simulink model "TosAeb"  (R2021a)
settings: Solver=FixedStepDiscrete  StartTime=0.0  StopTime=inf  FixedStep=0.01  RelTol=1e-3
undefined symbols (not defined in the file or in its folder): ACCModSts, AccSts1, AEB_ObjectMovingOrientation, AEB_ObjectMovingState, CN_BTCActive, CN_CurrentGearR, CN_CurrentGearR_DHT, CN_PTCActive, CN_VDCActive, CN_VSC_EspOff, CN_VSC_FCW_AEB_Error, CN_VSC_FCW_AEB_NoDispay, CN_VSC_FCW_AEB_PerfmDgdtn, CN_VSC_FCW_AEB_TempUnAvlbl, CN_VSC_VehStandstill, CNaccelAvoidMax, CNaccelAvoidMin, CNbrakePedalPress, CNcomfortStrLeftAccelMax, CNcomfortStrLeftAccelMin, CNcomfortStrRightAccelMax, CNcomfortStrRightAccelMin, CNDrvDoorOpen, CNDrvModeEco, CNDrvModeNorm, CNDrvModeOther, CNDrvModeSport, CNDrvSBRreminder, CNNull, CNobjInvalidID, CNPassDoorOpen, CNPassSBRreminder, CNPEBSAccelNegInf, CNPEBSAccelNull, CNPEBSTimeInf, CNPEBSTimeNull, CNSTOPMAX, CNSTOPNULL, CNsubjWidth, CNSwtOFF, CNSwtON, CNTTCMAX, CNTTCNULL, CNVehConfigAEBCycNone, CNVehConfigAEBNone, CNVehConfigAEBPedNone, CNVehConfigFCWNone, CNVehConfigJANone, CollisionSeverity, FALSE, FCWSnvty, JACycObjCalcInfoDefaultParams, JAPedObjCalcInfoDefaultParams, JAVehObjCalcInfoDefaultParams, latCycObjCalcInfoDefaultParams, latPedObjCalcInfoDefaultParams, longObjCalcInfoDefaultParams, ModSts, movingStatus, ObjectType ... (+1112)
libraries: simulink x3031, AEBSFunctionLib x50, Ta_Library x33, AEBSStateMachineLib x23, Mylibrary x12, simulink_extras x7, ObjSelect_lib x3, dspstat3 x2
(part 45 of 102)

== system TosAeb/FcwAebJa_Step_sys/ALG_AEBSystem/ALG_AEBController/AEB_FuncImpManagementApp/FuncImpArbCalc/BrkFuncImpArbCalc/BrkFuncImpSuppCondCalc/AEBpartFuncImpSuppCondCalc ==
  AEBfullModStsArb : Inport
  pedAEBfullModStsArb : Inport  Port=2
  cycAEBpartModStsArb : Inport  Port=3
  cycAEBfullModStsArb : Inport  Port=4
  JA_vehAEBfullModStsArb : Inport  Port=5
  EBAModStsArb : Inport  Port=6
  JA_PTW_AEBfullModStsArb : Inport  Port=7
  JA_cycAEBfullModStsArb : Inport  Port=8
  JA_pedAEBfullModStsArb : Inport  Port=9
  Compare To Constant{1..3,7..12} (9 alike) : simulink/Logic and Bit Operations/Compare To Constant  relop===  const=ModSts.ActiveState  OutDataTypeStr=boolean
  Logical Operator1 : Logic  Operator=OR  Inputs=9  AllPortsSameDT=off  OutDataTypeStr=boolean
  AEBpartFuncImpSuppCond : Outport
  wires:
  Compare To Constant3/1 -> Logical Operator1/9
  Compare To Constant2/1 -> Logical Operator1/8
  Compare To Constant1/1 -> Logical Operator1/7
  JA_pedAEBfullModStsArb/1 -> Compare To Constant3/1
  JA_cycAEBfullModStsArb/1 -> Compare To Constant2/1
  JA_PTW_AEBfullModStsArb/1 -> Compare To Constant1/1
  Logical Operator1/1 -> AEBpartFuncImpSuppCond/1
  Compare To Constant9/1 -> Logical Operator1/3
  Compare To Constant11/1 -> Logical Operator1/5
  pedAEBfullModStsArb/1 -> Compare To Constant8/1
  Compare To Constant10/1 -> Logical Operator1/4
  Compare To Constant7/1 -> Logical Operator1/1
  Compare To Constant8/1 -> Logical Operator1/2
  EBAModStsArb/1 -> Compare To Constant12/1
  AEBfullModStsArb/1 -> Compare To Constant7/1
  cycAEBpartModStsArb/1 -> Compare To Constant9/1
  cycAEBfullModStsArb/1 -> Compare To Constant10/1
  JA_vehAEBfullModStsArb/1 -> Compare To Constant11/1
  Compare To Constant12/1 -> Logical Operator1/6

== system TosAeb/FcwAebJa_Step_sys/ALG_AEBSystem/ALG_AEBController/AEB_FuncImpManagementApp/FuncImpArbCalc/BrkFuncImpArbCalc/BrkFuncImpSuppCondCalc/EBAFuncImpSuppCondCalc ==
  Constant : Constant  Value=FALSE
  EBAFuncImpSuppCond : Outport
  wires:
  Constant/1 -> EBAFuncImpSuppCond/1

== system TosAeb/FcwAebJa_Step_sys/ALG_AEBSystem/ALG_AEBController/AEB_FuncImpManagementApp/FuncImpArbCalc/BrkFuncImpArbCalc/BrkFuncImpSuppCondCalc/FCTBFuncImpSuppCondCalc ==
  AEBpartModStsArb : Inport
  AEBfullModStsArb : Inport  Port=2
  EBAModStsArb : Inport  Port=3
  pedAEBfullModStsArb : Inport  Port=4
  cycAEBfullModStsArb : Inport  Port=5
  cycAEBpartModStsArb : Inport  Port=6
  JA_vehAEBfullModStsArb : Inport  Port=7
  JA_PTW_AEBModStsArb : Inport  Port=8
  JA_cycAEBModStsArb : Inport  Port=9
  JA_pedAEBModStsArb : Inport  Port=10
  Compare To Constant{,1..9} (10 alike) : simulink/Logic and Bit Operations/Compare To Constant  relop===  const=ModSts.ActiveState  OutDataTypeStr=boolean
  Logical Operator : Logic  Operator=OR  Inputs=10  AllPortsSameDT=off  OutDataTypeStr=boolean
  FCTBFuncImpSuppCond : Outport
  wires:
  JA_pedAEBModStsArb/1 -> Compare To Constant9/1
  Compare To Constant9/1 -> Logical Operator/10
  JA_cycAEBModStsArb/1 -> Compare To Constant8/1
  Compare To Constant8/1 -> Logical Operator/9
  Compare To Constant7/1 -> Logical Operator/8
  JA_PTW_AEBModStsArb/1 -> Compare To Constant7/1
  Compare To Constant2/1 -> Logical Operator/3
  Compare To Constant5/1 -> Logical Operator/6
  Compare To Constant/1 -> Logical Operator/1
  Compare To Constant4/1 -> Logical Operator/5
  EBAModStsArb/1 -> Compare To Constant2/1
  Compare To Constant6/1 -> Logical Operator/7
  Compare To Constant3/1 -> Logical Operator/4
  Logical Operator/1 -> FCTBFuncImpSuppCond/1
  Compare To Constant1/1 -> Logical Operator/2
  pedAEBfullModStsArb/1 -> Compare To Constant3/1
  cycAEBfullModStsArb/1 -> Compare To Constant4/1
  AEBfullModStsArb/1 -> Compare To Constant1/1
  AEBpartModStsArb/1 -> Compare To Constant/1
  JA_vehAEBfullModStsArb/1 -> Compare To Constant6/1
  cycAEBpartModStsArb/1 -> Compare To Constant5/1

== system TosAeb/FcwAebJa_Step_sys/ALG_AEBSystem/ALG_AEBController/AEB_FuncImpManagementApp/FuncImpArbCalc/BrkFuncImpArbCalc/BrkFuncImpSuppCondCalc/JA_PTW_AEBfullFuncImpSuppCondCalc ==
  AEBfullModStsArb : Inport
  pedAEBfullModStsArb : Inport  Port=2
  JA_pedAEBfullModStsArb : Inport  Port=3
  cycAEBfullModStsArb : Inport  Port=4
  EBAModStsArb : Inport  Port=5
  Compare To Constant{7..10,12} (5 alike) : simulink/Logic and Bit Operations/Compare To Constant  relop===  const=ModSts.ActiveState  OutDataTypeStr=boolean
  Logical Operator1 : Logic  Operator=OR  Inputs=5  AllPortsSameDT=off  OutDataTypeStr=boolean
  JA_PTW_AEBfullFuncImpSuppCond : Outport
  wires:
  Compare To Constant12/1 -> Logical Operator1/5
  cycAEBfullModStsArb/1 -> Compare To Constant10/1
  JA_pedAEBfullModStsArb/1 -> Compare To Constant9/1
  AEBfullModStsArb/1 -> Compare To Constant7/1
  EBAModStsArb/1 -> Compare To Constant12/1
  Compare To Constant8/1 -> Logical Operator1/2
  Compare To Constant7/1 -> Logical Operator1/1
  Compare To Constant10/1 -> Logical Operator1/4
  pedAEBfullModStsArb/1 -> Compare To Constant8/1
  Compare To Constant9/1 -> Logical Operator1/3
  Logical Operator1/1 -> JA_PTW_AEBfullFuncImpSuppCond/1

== system TosAeb/FcwAebJa_Step_sys/ALG_AEBSystem/ALG_AEBController/AEB_FuncImpManagementApp/FuncImpArbCalc/BrkFuncImpArbCalc/BrkFuncImpSuppCondCalc/JA_cycAEBfullFuncImpSuppCondCalc ==
  cycAEBfullModStsArb : Inport
  pedAEBfullModStsArb : Inport  Port=2
  EBAModStsArb : Inport  Port=3
  JA_pedAEBModStsArb : Inport  Port=4
  Compare To Constant{1,19..21} (4 alike) : simulink/Logic and Bit Operations/Compare To Constant  relop===  const=ModSts.ActiveState  OutDataTypeStr=boolean
  Logical Operator3 : Logic  Operator=OR  Inputs=4  AllPortsSameDT=off  OutDataTypeStr=boolean
  JA_cycAEBfullFuncImpSuppCond : Outport
  wires:
  Compare To Constant1/1 -> Logical Operator3/4
  JA_pedAEBModStsArb/1 -> Compare To Constant1/1
  Compare To Constant19/1 -> Logical Operator3/1
  Compare To Constant21/1 -> Logical Operator3/3
  Compare To Constant20/1 -> Logical Operator3/2
  pedAEBfullModStsArb/1 -> Compare To Constant20/1
  EBAModStsArb/1 -> Compare To Constant21/1
  Logical Operator3/1 -> JA_cycAEBfullFuncImpSuppCond/1
  cycAEBfullModStsArb/1 -> Compare To Constant19/1

== system TosAeb/FcwAebJa_Step_sys/ALG_AEBSystem/ALG_AEBController/AEB_FuncImpManagementApp/FuncImpArbCalc/BrkFuncImpArbCalc/BrkFuncImpSuppCondCalc/JA_pedAEBfullFuncImpSuppCondCalc ==
  cycAEBfullModStsArb : Inport
  pedAEBfullModStsArb : Inport  Port=2
  EBAModStsArb : Inport  Port=3
  Compare To Constant19 : simulink/Logic and Bit Operations/Compare To Constant  relop===  const=ModSts.ActiveState  OutDataTypeStr=boolean
  Compare To Constant20 : simulink/Logic and Bit Operations/Compare To Constant  relop===  const=ModSts.ActiveState  OutDataTypeStr=boolean
  Compare To Constant21 : simulink/Logic and Bit Operations/Compare To Constant  relop===  const=ModSts.ActiveState  OutDataTypeStr=boolean
  Logical Operator3 : Logic  Operator=OR  Inputs=3  AllPortsSameDT=off  OutDataTypeStr=boolean
  JA_pedAEBfullFuncImpSuppCond : Outport
  wires:
  cycAEBfullModStsArb/1 -> Compare To Constant19/1
  Logical Operator3/1 -> JA_pedAEBfullFuncImpSuppCond/1
  EBAModStsArb/1 -> Compare To Constant21/1
  pedAEBfullModStsArb/1 -> Compare To Constant20/1
  Compare To Constant20/1 -> Logical Operator3/2
  Compare To Constant21/1 -> Logical Operator3/3
  Compare To Constant19/1 -> Logical Operator3/1

== system TosAeb/FcwAebJa_Step_sys/ALG_AEBSystem/ALG_AEBController/AEB_FuncImpManagementApp/FuncImpArbCalc/BrkFuncImpArbCalc/BrkFuncImpSuppCondCalc/JA_vehAEBfullFuncImpSuppCondCalc ==
  AEBfullModStsArb : Inport
  cycAEBfullModStsArb : Inport  Port=2
  pedAEBfullModStsArb : Inport  Port=3
  EBAModStsArb : Inport  Port=4
  JA_PTW_AEBModStsArb : Inport  Port=5
  JA_cycAEBModStsArb : Inport  Port=6
  JA_pedAEBModStsArb : Inport  Port=7
  Compare To Constant{1..3,18..21} (7 alike) : simulink/Logic and Bit Operations/Compare To Constant  relop===  const=ModSts.ActiveState  OutDataTypeStr=boolean
  Logical Operator3 : Logic  Operator=OR  Inputs=7  AllPortsSameDT=off  OutDataTypeStr=boolean
  JA_vehAEBfullFuncImpSuppCond : Outport
  wires:
  Compare To Constant3/1 -> Logical Operator3/7
  Compare To Constant2/1 -> Logical Operator3/6
  Compare To Constant1/1 -> Logical Operator3/5
  JA_pedAEBModStsArb/1 -> Compare To Constant3/1
  JA_cycAEBModStsArb/1 -> Compare To Constant2/1
  JA_PTW_AEBModStsArb/1 -> Compare To Constant1/1
  Compare To Constant19/1 -> Logical Operator3/2
  Compare To Constant21/1 -> Logical Operator3/4
  Compare To Constant20/1 -> Logical Operator3/3
  AEBfullModStsArb/1 -> Compare To Constant18/1
  pedAEBfullModStsArb/1 -> Compare To Constant20/1
  EBAModStsArb/1 -> Compare To Constant21/1
  Logical Operator3/1 -> JA_vehAEBfullFuncImpSuppCond/1
  Compare To Constant18/1 -> Logical Operator3/1
  cycAEBfullModStsArb/1 -> Compare To Constant19/1

== system TosAeb/FcwAebJa_Step_sys/ALG_AEBSystem/ALG_AEBController/AEB_FuncImpManagementApp/FuncImpArbCalc/BrkFuncImpArbCalc/BrkFuncImpSuppCondCalc/cycAEBfullFuncImpSuppCondCalc ==
  pedAEBfullModStsArb : Inport
  EBAModStsArb : Inport  Port=2
  Compare To Constant22 : simulink/Logic and Bit Operations/Compare To Constant  relop===  const=ModSts.ActiveState  OutDataTypeStr=boolean
  Compare To Constant26 : simulink/Logic and Bit Operations/Compare To Constant  relop===  const=ModSts.ActiveState  OutDataTypeStr=boolean
  Logical Operator5 : Logic  Operator=OR  AllPortsSameDT=off  OutDataTypeStr=boolean
  cycAEBfullFuncImpSuppCond : Outport
  wires:
  EBAModStsArb/1 -> Compare To Constant26/1
  Compare To Constant26/1 -> Logical Operator5/2
  Compare To Constant22/1 -> Logical Operator5/1
  pedAEBfullModStsArb/1 -> Compare To Constant22/1
  Logical Operator5/1 -> cycAEBfullFuncImpSuppCond/1

== system TosAeb/FcwAebJa_Step_sys/ALG_AEBSystem/ALG_AEBController/AEB_FuncImpManagementApp/FuncImpArbCalc/BrkFuncImpArbCalc/BrkFuncImpSuppCondCalc/cycAEBpartFuncImpSuppCondCalc ==
  AEBfullModStsArb : Inport
  pedAEBfullModStsArb : Inport  Port=2
  cycAEBfullModStsArb : Inport  Port=3
  JA_vehAEBfullModStsArb : Inport  Port=4
  EBAModStsArb : Inport  Port=5
  JA_PTW_AEBfullModStsArb : Inport  Port=6
  JA_cycAEBfullModStsArb : Inport  Port=7
  JA_pedAEBfullModStsArb : Inport  Port=8
  Compare To Constant{1..3,13..17} (8 alike) : simulink/Logic and Bit Operations/Compare To Constant  relop===  const=ModSts.ActiveState  OutDataTypeStr=boolean
  Logical Operator2 : Logic  Operator=OR  Inputs=8  AllPortsSameDT=off  OutDataTypeStr=boolean
  cycAEBpartFuncImpSuppCond : Outport
  wires:
  Compare To Constant3/1 -> Logical Operator2/8
  Compare To Constant2/1 -> Logical Operator2/7
  Compare To Constant1/1 -> Logical Operator2/6
  JA_pedAEBfullModStsArb/1 -> Compare To Constant3/1
  JA_cycAEBfullModStsArb/1 -> Compare To Constant2/1
  JA_PTW_AEBfullModStsArb/1 -> Compare To Constant1/1
  pedAEBfullModStsArb/1 -> Compare To Constant14/1
  Compare To Constant17/1 -> Logical Operator2/5
  Compare To Constant13/1 -> Logical Operator2/1
  Compare To Constant15/1 -> Logical Operator2/3
  Compare To Constant16/1 -> Logical Operator2/4
  JA_vehAEBfullModStsArb/1 -> Compare To Constant16/1
  Compare To Constant14/1 -> Logical Operator2/2
  Logical Operator2/1 -> cycAEBpartFuncImpSuppCond/1
  EBAModStsArb/1 -> Compare To Constant17/1
  AEBfullModStsArb/1 -> Compare To Constant13/1
  cycAEBfullModStsArb/1 -> Compare To Constant15/1

== system TosAeb/FcwAebJa_Step_sys/ALG_AEBSystem/ALG_AEBController/AEB_FuncImpManagementApp/FuncImpArbCalc/BrkFuncImpArbCalc/BrkFuncImpSuppCondCalc/pedAEBfullFuncImpSuppCondCalc ==
  EBAModStsArb : Inport
  Compare To Constant27 : simulink/Logic and Bit Operations/Compare To Constant  relop===  const=ModSts.ActiveState  OutDataTypeStr=boolean
  pedAEBfullFuncImpSuppCond : Outport
  wires:
  EBAModStsArb/1 -> Compare To Constant27/1
  Compare To Constant27/1 -> pedAEBfullFuncImpSuppCond/1

== system TosAeb/FcwAebJa_Step_sys/ALG_AEBSystem/ALG_AEBController/AEB_FuncImpManagementApp/FuncImpArbCalc/BrkFuncImpArbCalc/BrkFuncImpSuppCondCalc1/AEBfullFuncImpSuppCondCalc ==
  pedAEBfullModStsArb : Inport
  cycAEBfullModStsArb : Inport  Port=2
  EBAModStsArb : Inport  Port=3
  Compare To Constant23 : simulink/Logic and Bit Operations/Compare To Constant  relop===  const=ModSts.ActiveState  OutDataTypeStr=boolean
  Compare To Constant24 : simulink/Logic and Bit Operations/Compare To Constant  relop===  const=ModSts.ActiveState  OutDataTypeStr=boolean
  Compare To Constant25 : simulink/Logic and Bit Operations/Compare To Constant  relop===  const=ModSts.ActiveState  OutDataTypeStr=boolean
  Logical Operator4 : Logic  Operator=OR  Inputs=3  AllPortsSameDT=off  OutDataTypeStr=boolean
  AEBfullFuncImpSuppCond : Outport
  wires:
  Compare To Constant23/1 -> Logical Operator4/1
  Compare To Constant24/1 -> Logical Operator4/2
  pedAEBfullModStsArb/1 -> Compare To Constant23/1
  EBAModStsArb/1 -> Compare To Constant25/1
  cycAEBfullModStsArb/1 -> Compare To Constant24/1
  Logical Operator4/1 -> AEBfullFuncImpSuppCond/1
  Compare To Constant25/1 -> Logical Operator4/3

== system TosAeb/FcwAebJa_Step_sys/ALG_AEBSystem/ALG_AEBController/AEB_FuncImpManagementApp/FuncImpArbCalc/BrkFuncImpArbCalc/BrkFuncImpSuppCondCalc1/AEBpartFuncImpSuppCondCalc ==
  AEBfullModStsArb : Inport
  pedAEBfullModStsArb : Inport  Port=2
  cycAEBpartModStsArb : Inport  Port=3
  cycAEBfullModStsArb : Inport  Port=4
  JA_vehAEBfullModStsArb : Inport  Port=5
  EBAModStsArb : Inport  Port=6
  Compare To Constant{7..12} (6 alike) : simulink/Logic and Bit Operations/Compare To Constant  relop===  const=ModSts.ActiveState  OutDataTypeStr=boolean
  Logical Operator1 : Logic  Operator=OR  Inputs=6  AllPortsSameDT=off  OutDataTypeStr=boolean
  AEBpartFuncImpSuppCond : Outport
  wires:
  Logical Operator1/1 -> AEBpartFuncImpSuppCond/1
  EBAModStsArb/1 -> Compare To Constant12/1
  Compare To Constant12/1 -> Logical Operator1/6
  Compare To Constant10/1 -> Logical Operator1/4
  AEBfullModStsArb/1 -> Compare To Constant7/1
  Compare To Constant7/1 -> Logical Operator1/1
  pedAEBfullModStsArb/1 -> Compare To Constant8/1
  Compare To Constant8/1 -> Logical Operator1/2
  Compare To Constant9/1 -> Logical Operator1/3
  cycAEBfullModStsArb/1 -> Compare To Constant10/1
  JA_vehAEBfullModStsArb/1 -> Compare To Constant11/1
  cycAEBpartModStsArb/1 -> Compare To Constant9/1
  Compare To Constant11/1 -> Logical Operator1/5

== system TosAeb/FcwAebJa_Step_sys/ALG_AEBSystem/ALG_AEBController/AEB_FuncImpManagementApp/FuncImpArbCalc/BrkFuncImpArbCalc/BrkFuncImpSuppCondCalc1/EBAFuncImpSuppCondCalc ==
  Constant : Constant  Value=FALSE
  EBAFuncImpSuppCond : Outport
  wires:
  Constant/1 -> EBAFuncImpSuppCond/1

== system TosAeb/FcwAebJa_Step_sys/ALG_AEBSystem/ALG_AEBController/AEB_FuncImpManagementApp/FuncImpArbCalc/BrkFuncImpArbCalc/BrkFuncImpSuppCondCalc1/FCTBFuncImpSuppCondCalc ==
  AEBpartModStsArb : Inport
  AEBfullModStsArb : Inport  Port=2
  EBAModStsArb : Inport  Port=3
  pedAEBfullModStsArb : Inport  Port=4
  cycAEBfullModStsArb : Inport  Port=5
  cycAEBpartModStsArb : Inport  Port=6
  JA_vehAEBfullModStsArb : Inport  Port=7
  Compare To Constant{,1..6} (7 alike) : simulink/Logic and Bit Operations/Compare To Constant  relop===  const=ModSts.ActiveState  OutDataTypeStr=boolean
  Logical Operator : Logic  Operator=OR  Inputs=7  AllPortsSameDT=off  OutDataTypeStr=boolean
  FCTBFuncImpSuppCond : Outport
  wires:
  Compare To Constant2/1 -> Logical Operator/3
  Compare To Constant6/1 -> Logical Operator/7
  Compare To Constant3/1 -> Logical Operator/4
  Compare To Constant5/1 -> Logical Operator/6
  Compare To Constant/1 -> Logical Operator/1
  AEBfullModStsArb/1 -> Compare To Constant1/1
  AEBpartModStsArb/1 -> Compare To Constant/1
  Logical Operator/1 -> FCTBFuncImpSuppCond/1
  Compare To Constant4/1 -> Logical Operator/5
  Compare To Constant1/1 -> Logical Operator/2
  EBAModStsArb/1 -> Compare To Constant2/1
  cycAEBfullModStsArb/1 -> Compare To Constant4/1
  pedAEBfullModStsArb/1 -> Compare To Constant3/1
  JA_vehAEBfullModStsArb/1 -> Compare To Constant6/1
  cycAEBpartModStsArb/1 -> Compare To Constant5/1

== system TosAeb/FcwAebJa_Step_sys/ALG_AEBSystem/ALG_AEBController/AEB_FuncImpManagementApp/FuncImpArbCalc/BrkFuncImpArbCalc/BrkFuncImpSuppCondCalc1/JA_vehAEBfullFuncImpSuppCondCalc ==
  AEBfullModStsArb : Inport
  cycAEBfullModStsArb : Inport  Port=2
  pedAEBfullModStsArb : Inport  Port=3
  EBAModStsArb : Inport  Port=4
  Compare To Constant{18..21} (4 alike) : simulink/Logic and Bit Operations/Compare To Constant  relop===  const=ModSts.ActiveState  OutDataTypeStr=boolean
  Logical Operator3 : Logic  Operator=OR  Inputs=4  AllPortsSameDT=off  OutDataTypeStr=boolean
  JA_vehAEBfullFuncImpSuppCond : Outport
  wires:
  Logical Operator3/1 -> JA_vehAEBfullFuncImpSuppCond/1
  AEBfullModStsArb/1 -> Compare To Constant18/1
  cycAEBfullModStsArb/1 -> Compare To Constant19/1
  Compare To Constant19/1 -> Logical Operator3/2
  Compare To Constant20/1 -> Logical Operator3/3
  EBAModStsArb/1 -> Compare To Constant21/1
  pedAEBfullModStsArb/1 -> Compare To Constant20/1
  Compare To Constant21/1 -> Logical Operator3/4
  Compare To Constant18/1 -> Logical Operator3/1

== system TosAeb/FcwAebJa_Step_sys/ALG_AEBSystem/ALG_AEBController/AEB_FuncImpManagementApp/FuncImpArbCalc/BrkFuncImpArbCalc/BrkFuncImpSuppCondCalc1/cycAEBfullFuncImpSuppCondCalc ==
  <pedAEBfullModStsArb> : Inport
  <EBAModStsArb> : Inport  Port=2
  Compare To Constant22 : simulink/Logic and Bit Operations/Compare To Constant  relop===  const=ModSts.ActiveState  OutDataTypeStr=boolean
  Compare To Constant26 : simulink/Logic and Bit Operations/Compare To Constant  relop===  const=ModSts.ActiveState  OutDataTypeStr=boolean
  Logical Operator5 : Logic  Operator=OR  AllPortsSameDT=off  OutDataTypeStr=boolean
  cycAEBfullFuncImpSuppCond1 : Outport
  wires:
  <EBAModStsArb>/1 -> Compare To Constant26/1
  Logical Operator5/1 -> cycAEBfullFuncImpSuppCond1/1
  Compare To Constant22/1 -> Logical Operator5/1
  Compare To Constant26/1 -> Logical Operator5/2
  <pedAEBfullModStsArb>/1 -> Compare To Constant22/1

== system TosAeb/FcwAebJa_Step_sys/ALG_AEBSystem/ALG_AEBController/AEB_FuncImpManagementApp/FuncImpArbCalc/BrkFuncImpArbCalc/BrkFuncImpSuppCondCalc1/cycAEBpartFuncImpSuppCondCalc ==
  <AEBfullModStsArb> : Inport
  <pedAEBfullModStsArb> : Inport  Port=2
  <cycAEBfullModStsArb> : Inport  Port=3
  <JA_vehAEBfullModStsArb> : Inport  Port=4
  <EBAModStsArb> : Inport  Port=5
  Compare To Constant{13..17} (5 alike) : simulink/Logic and Bit Operations/Compare To Constant  relop===  const=ModSts.ActiveState  OutDataTypeStr=boolean
  Logical Operator2 : Logic  Operator=OR  Inputs=5  AllPortsSameDT=off  OutDataTypeStr=boolean
  cycAEBpartFuncImpSuppCond : Outport
  wires:
  Compare To Constant14/1 -> Logical Operator2/2
  Compare To Constant13/1 -> Logical Operator2/1
  Compare To Constant15/1 -> Logical Operator2/3
  Compare To Constant16/1 -> Logical Operator2/4
  Logical Operator2/1 -> cycAEBpartFuncImpSuppCond/1
  <JA_vehAEBfullModStsArb>/1 -> Compare To Constant16/1
  <EBAModStsArb>/1 -> Compare To Constant17/1
  <pedAEBfullModStsArb>/1 -> Compare To Constant14/1
  <AEBfullModStsArb>/1 -> Compare To Constant13/1
  <cycAEBfullModStsArb>/1 -> Compare To Constant15/1
  Compare To Constant17/1 -> Logical Operator2/5

== system TosAeb/FcwAebJa_Step_sys/ALG_AEBSystem/ALG_AEBController/AEB_FuncImpManagementApp/FuncImpArbCalc/BrkFuncImpArbCalc/BrkFuncImpSuppCondCalc1/pedAEBfullFuncImpSuppCondCalc ==
  EBAModStsArb : Inport
  Compare To Constant27 : simulink/Logic and Bit Operations/Compare To Constant  relop===  const=ModSts.ActiveState  OutDataTypeStr=boolean
  pedAEBfullFuncImpSuppCond : Outport
  wires:
  EBAModStsArb/1 -> Compare To Constant27/1
  Compare To Constant27/1 -> pedAEBfullFuncImpSuppCond/1

== system TosAeb/FcwAebJa_Step_sys/ALG_AEBSystem/ALG_AEBController/AEB_FuncImpManagementApp/FuncImpArbCalc/BrkFuncImpArbCalc1/BrkFuncImpAborCondCalc/AEBfullFuncImpAborCondCalc ==
  AEBModStsArb : Inport
  Bus Selector4 : BusSelector  OutputSignals=latAEBModStsArb.pedAEBfullModStsArb,latAEBModStsArb.cycAEBfullModStsArb,vehAEBModStsArb.EBAModStsArb
  Compare To Constant23 : simulink/Logic and Bit Operations/Compare To Constant  relop===  const=ModSts.ActiveState  OutDataTypeStr=boolean
  Compare To Constant24 : simulink/Logic and Bit Operations/Compare To Constant  relop===  const=ModSts.ActiveState  OutDataTypeStr=boolean
  Compare To Constant25 : simulink/Logic and Bit Operations/Compare To Constant  relop===  const=ModSts.ActiveState  OutDataTypeStr=boolean
  Logical Operator4 : Logic  Operator=OR  Inputs=3  AllPortsSameDT=off  OutDataTypeStr=boolean
  AEBfullFuncImpAborCond : Outport
  wires:
  AEBModStsArb/1 -> Bus Selector4/1
  Compare To Constant24/1 -> Logical Operator4/2
  Compare To Constant23/1 -> Logical Operator4/1
  Compare To Constant25/1 -> Logical Operator4/3
  Logical Operator4/1 -> AEBfullFuncImpAborCond/1
  Bus Selector4/1 -> Compare To Constant23/1  "<pedAEBfullModStsArb>"
  Bus Selector4/3 -> Compare To Constant25/1  "<EBAModStsArb>"
  Bus Selector4/2 -> Compare To Constant24/1  "<cycAEBfullModStsArb>"

== system TosAeb/FcwAebJa_Step_sys/ALG_AEBSystem/ALG_AEBController/AEB_FuncImpManagementApp/FuncImpArbCalc/BrkFuncImpArbCalc1/BrkFuncImpAborCondCalc/AEBpartFuncImpAborCondCalc ==
  AEBModStsArb : Inport
  Bus Selector1 : BusSelector  OutputSignals=vehAEBModStsArb.AEBfullModStsArb,latAEBModStsArb.pedAEBfullModStsArb,latAEBModStsArb.cycAEBpartModStsArb,latAEBModStsArb.cycAEBfullModStsArb,JA_vehAEBModStsArb.JA_vehAEBfullModStsArb,vehAEBModStsArb.EBAModStsArb
  Compare To Constant{7..12} (6 alike) : simulink/Logic and Bit Operations/Compare To Constant  relop===  const=ModSts.ActiveState  OutDataTypeStr=boolean
  Logical Operator1 : Logic  Operator=OR  Inputs=6  AllPortsSameDT=off  OutDataTypeStr=boolean
  AEBpartFuncImpAborCond : Outport
  wires:
  AEBModStsArb/1 -> Bus Selector1/1
  Bus Selector1/6 -> Compare To Constant12/1  "<EBAModStsArb>"
  Logical Operator1/1 -> AEBpartFuncImpAborCond/1
  Compare To Constant12/1 -> Logical Operator1/6
  Compare To Constant7/1 -> Logical Operator1/1
  Compare To Constant11/1 -> Logical Operator1/5
  Compare To Constant8/1 -> Logical Operator1/2
  Compare To Constant10/1 -> Logical Operator1/4
  Compare To Constant9/1 -> Logical Operator1/3
  Bus Selector1/5 -> Compare To Constant11/1  "<JA_vehAEBfullModStsArb>"
  Bus Selector1/1 -> Compare To Constant7/1  "<AEBfullModStsArb>"
  Bus Selector1/3 -> Compare To Constant9/1  "<cycAEBpartModStsArb>"
  Bus Selector1/4 -> Compare To Constant10/1  "<cycAEBfullModStsArb>"
  Bus Selector1/2 -> Compare To Constant8/1  "<pedAEBfullModStsArb>"

== system TosAeb/FcwAebJa_Step_sys/ALG_AEBSystem/ALG_AEBController/AEB_FuncImpManagementApp/FuncImpArbCalc/BrkFuncImpArbCalc1/BrkFuncImpAborCondCalc/EBAFuncImpAborCondCalc ==
  Constant : Constant  Value=FALSE
  EBAFuncImpAborCond : Outport
  wires:
  Constant/1 -> EBAFuncImpAborCond/1

== system TosAeb/FcwAebJa_Step_sys/ALG_AEBSystem/ALG_AEBController/AEB_FuncImpManagementApp/FuncImpArbCalc/BrkFuncImpArbCalc1/BrkFuncImpAborCondCalc/FCTBFuncImpAborCondCalc ==
  AEBModStsArb : Inport
  Bus Selector : BusSelector  OutputSignals=vehAEBModStsArb.AEBpartModStsArb,vehAEBModStsArb.AEBfullModStsArb,vehAEBModStsArb.EBAModStsArb,latAEBModStsArb.pedAEBfullModStsArb,latAEBModStsArb.cycAEBfullModStsArb,latAEBModStsArb.cycAEBpartModStsArb,JA_vehAEBModStsArb.JA_vehAEBfullModStsArb
  Compare To Constant{,1..6} (7 alike) : simulink/Logic and Bit Operations/Compare To Constant  relop===  const=ModSts.ActiveState  OutDataTypeStr=boolean
  Logical Operator : Logic  Operator=OR  Inputs=7  AllPortsSameDT=off  OutDataTypeStr=boolean
  FCTBFuncImpAborCond : Outport
  wires:
  AEBModStsArb/1 -> Bus Selector/1
  Bus Selector/3 -> Compare To Constant2/1  "<EBAModStsArb>"
  Bus Selector/4 -> Compare To Constant3/1  "<pedAEBfullModStsArb>"
  Bus Selector/7 -> Compare To Constant6/1  "<JA_vehAEBfullModStsArb>"
  Bus Selector/5 -> Compare To Constant4/1  "<cycAEBfullModStsArb>"
  Bus Selector/6 -> Compare To Constant5/1  "<cycAEBpartModStsArb>"
  Compare To Constant3/1 -> Logical Operator/4
  Compare To Constant/1 -> Logical Operator/1
  Compare To Constant1/1 -> Logical Operator/2
  Compare To Constant6/1 -> Logical Operator/7
  Compare To Constant4/1 -> Logical Operator/5
  Logical Operator/1 -> FCTBFuncImpAborCond/1
  Compare To Constant5/1 -> Logical Operator/6
  Compare To Constant2/1 -> Logical Operator/3
  Bus Selector/2 -> Compare To Constant1/1  "<AEBfullModStsArb>"
  Bus Selector/1 -> Compare To Constant/1  "<AEBpartModStsArb>"

== system TosAeb/FcwAebJa_Step_sys/ALG_AEBSystem/ALG_AEBController/AEB_FuncImpManagementApp/FuncImpArbCalc/BrkFuncImpArbCalc1/BrkFuncImpAborCondCalc/JA_vehAEBfullFuncImpAborCondCalc ==
  AEBModStsArb : Inport
  Bus Selector3 : BusSelector  OutputSignals=vehAEBModStsArb.AEBfullModStsArb,latAEBModStsArb.cycAEBfullModStsArb,latAEBModStsArb.pedAEBfullModStsArb,vehAEBModStsArb.EBAModStsArb
  Compare To Constant{18..21} (4 alike) : simulink/Logic and Bit Operations/Compare To Constant  relop===  const=ModSts.ActiveState  OutDataTypeStr=boolean
  Logical Operator3 : Logic  Operator=OR  Inputs=4  AllPortsSameDT=off  OutDataTypeStr=boolean
  JA_vehAEBfullFuncImpAborCond : Outport
  wires:
  Compare To Constant21/1 -> Logical Operator3/4
  Compare To Constant18/1 -> Logical Operator3/1
  Compare To Constant19/1 -> Logical Operator3/2
  Logical Operator3/1 -> JA_vehAEBfullFuncImpAborCond/1
  Compare To Constant20/1 -> Logical Operator3/3
  Bus Selector3/2 -> Compare To Constant19/1  "<cycAEBfullModStsArb>"
  Bus Selector3/4 -> Compare To Constant21/1  "<EBAModStsArb>"
  Bus Selector3/3 -> Compare To Constant20/1  "<pedAEBfullModStsArb>"
  Bus Selector3/1 -> Compare To Constant18/1  "<AEBfullModStsArb>"
  AEBModStsArb/1 -> Bus Selector3/1

== system TosAeb/FcwAebJa_Step_sys/ALG_AEBSystem/ALG_AEBController/AEB_FuncImpManagementApp/FuncImpArbCalc/BrkFuncImpArbCalc1/BrkFuncImpAborCondCalc/cycAEBfullFuncImpAborCondCalc ==
  AEBModStsArb : Inport
  Bus Selector5 : BusSelector  OutputSignals=latAEBModStsArb.pedAEBfullModStsArb,vehAEBModStsArb.EBAModStsArb
  Compare To Constant22 : simulink/Logic and Bit Operations/Compare To Constant  relop===  const=ModSts.ActiveState  OutDataTypeStr=boolean
  Compare To Constant26 : simulink/Logic and Bit Operations/Compare To Constant  relop===  const=ModSts.ActiveState  OutDataTypeStr=boolean
  Logical Operator5 : Logic  Operator=OR  AllPortsSameDT=off  OutDataTypeStr=boolean
  cycAEBfullFuncImpAborCond : Outport
  wires:
  AEBModStsArb/1 -> Bus Selector5/1
  Bus Selector5/2 -> Compare To Constant26/1  "<EBAModStsArb>"
  Compare To Constant22/1 -> Logical Operator5/1
  Logical Operator5/1 -> cycAEBfullFuncImpAborCond/1
  Bus Selector5/1 -> Compare To Constant22/1  "<pedAEBfullModStsArb>"
  Compare To Constant26/1 -> Logical Operator5/2

== system TosAeb/FcwAebJa_Step_sys/ALG_AEBSystem/ALG_AEBController/AEB_FuncImpManagementApp/FuncImpArbCalc/BrkFuncImpArbCalc1/BrkFuncImpAborCondCalc/cycAEBpartFuncImpAborCondCalc ==
  AEBModStsArb : Inport
  Bus Selector2 : BusSelector  OutputSignals=vehAEBModStsArb.AEBfullModStsArb,latAEBModStsArb.pedAEBfullModStsArb,latAEBModStsArb.cycAEBfullModStsArb,JA_vehAEBModStsArb.JA_vehAEBfullModStsArb,vehAEBModStsArb.EBAModStsArb
  Compare To Constant{13..17} (5 alike) : simulink/Logic and Bit Operations/Compare To Constant  relop===  const=ModSts.ActiveState  OutDataTypeStr=boolean
  Logical Operator2 : Logic  Operator=OR  Inputs=5  AllPortsSameDT=off  OutDataTypeStr=boolean
  cycAEBpartFuncImpAborCond : Outport
  wires:
  Compare To Constant14/1 -> Logical Operator2/2
  Logical Operator2/1 -> cycAEBpartFuncImpAborCond/1
  AEBModStsArb/1 -> Bus Selector2/1
  Bus Selector2/5 -> Compare To Constant17/1  "<EBAModStsArb>"
  Bus Selector2/4 -> Compare To Constant16/1  "<JA_vehAEBfullModStsArb>"
  Compare To Constant13/1 -> Logical Operator2/1
  Compare To Constant15/1 -> Logical Operator2/3
  Compare To Constant16/1 -> Logical Operator2/4
  Compare To Constant17/1 -> Logical Operator2/5
  Bus Selector2/2 -> Compare To Constant14/1  "<pedAEBfullModStsArb>"
  Bus Selector2/3 -> Compare To Constant15/1  "<cycAEBfullModStsArb>"
  Bus Selector2/1 -> Compare To Constant13/1  "<AEBfullModStsArb>"

== system TosAeb/FcwAebJa_Step_sys/ALG_AEBSystem/ALG_AEBController/AEB_FuncImpManagementApp/FuncImpArbCalc/BrkFuncImpArbCalc1/BrkFuncImpAborCondCalc/pedAEBfullFuncImpAborCondCalc ==
  AEBModStsArb : Inport
  Bus Selector6 : BusSelector  OutputSignals=vehAEBModStsArb.EBAModStsArb
  Compare To Constant27 : simulink/Logic and Bit Operations/Compare To Constant  relop===  const=ModSts.ActiveState  OutDataTypeStr=boolean
  pedAEBfullFuncImpAborCond : Outport
  wires:
  AEBModStsArb/1 -> Bus Selector6/1
  Compare To Constant27/1 -> pedAEBfullFuncImpAborCond/1
  Bus Selector6/1 -> Compare To Constant27/1  "<EBAModStsArb>"

== system TosAeb/FcwAebJa_Step_sys/ALG_AEBSystem/ALG_AEBController/AEB_FuncImpManagementApp/FuncImpArbCalc/BrkFuncImpArbCalc1/BrkFuncImpArbCalc/AEBfullFuncImpArbCalc ==
  AEBfullModStsArb : Inport
  AEBfullFuncImpAborCond : Inport  Port=2
  Compare To Constant : simulink/Logic and Bit Operations/Compare To Constant  relop=~=  const=ModSts.ActiveState  OutDataTypeStr=boolean
  Compare To Constant1 : simulink/Logic and Bit Operations/Compare To Constant  relop===  const=FALSE  OutDataTypeStr=boolean
  Constant : Constant  Value=ModSts.StandbyState
  Switch : Switch  Criteria=u2 ~= 0
  Switch1 : Switch  Criteria=u2 ~= 0
  AEBfullFuncImpArb : Outport
  wires:
  AEBfullModStsArb/1 -> Switch1/1, Switch/1, Compare To Constant/1
  Compare To Constant/1 -> Switch/2
  AEBfullFuncImpAborCond/1 -> Compare To Constant1/1
  Compare To Constant1/1 -> Switch1/2
  Constant/1 -> Switch1/3
  Switch1/1 -> Switch/3
  Switch/1 -> AEBfullFuncImpArb/1

== system TosAeb/FcwAebJa_Step_sys/ALG_AEBSystem/ALG_AEBController/AEB_FuncImpManagementApp/FuncImpArbCalc/BrkFuncImpArbCalc1/BrkFuncImpArbCalc/AEBpartFuncImpArbCalc ==
  AEBpartModStsArb : Inport
  AEBpartFuncImpAborCond : Inport  Port=2
  Compare To Constant : simulink/Logic and Bit Operations/Compare To Constant  relop=~=  const=ModSts.ActiveState  OutDataTypeStr=boolean
  Compare To Constant1 : simulink/Logic and Bit Operations/Compare To Constant  relop===  const=FALSE  OutDataTypeStr=boolean
  Constant : Constant  Value=ModSts.StandbyState
  Switch : Switch  Criteria=u2 ~= 0
  Switch1 : Switch  Criteria=u2 ~= 0
  AEBpartFuncImpArb : Outport
  wires:
  AEBpartModStsArb/1 -> Switch1/1, Switch/1, Compare To Constant/1
  Compare To Constant/1 -> Switch/2
  AEBpartFuncImpAborCond/1 -> Compare To Constant1/1
  Compare To Constant1/1 -> Switch1/2
  Constant/1 -> Switch1/3
  Switch1/1 -> Switch/3
  Switch/1 -> AEBpartFuncImpArb/1

== system TosAeb/FcwAebJa_Step_sys/ALG_AEBSystem/ALG_AEBController/AEB_FuncImpManagementApp/FuncImpArbCalc/BrkFuncImpArbCalc1/BrkFuncImpArbCalc/EBAFuncImpArbCalc ==
  EBAModStsArb : Inport
  EBAFuncImpAborCond : Inport  Port=2
  Compare To Constant : simulink/Logic and Bit Operations/Compare To Constant  relop=~=  const=ModSts.ActiveState  OutDataTypeStr=boolean
  Compare To Constant1 : simulink/Logic and Bit Operations/Compare To Constant  relop===  const=FALSE  OutDataTypeStr=boolean
  Constant : Constant  Value=ModSts.StandbyState
  Switch : Switch  Criteria=u2 ~= 0
  Switch1 : Switch  Criteria=u2 ~= 0
  EBAFuncImpArb : Outport
  wires:
  Switch/1 -> EBAFuncImpArb/1
  Switch1/1 -> Switch/3
  Constant/1 -> Switch1/3
  Compare To Constant1/1 -> Switch1/2
  EBAFuncImpAborCond/1 -> Compare To Constant1/1
  Compare To Constant/1 -> Switch/2
  EBAModStsArb/1 -> Compare To Constant/1, Switch/1, Switch1/1

== system TosAeb/FcwAebJa_Step_sys/ALG_AEBSystem/ALG_AEBController/AEB_FuncImpManagementApp/FuncImpArbCalc/BrkFuncImpArbCalc1/BrkFuncImpArbCalc/FCTBFuncImpArbCalc ==
  FCTBModStsArb : Inport
  FCTBFuncImpAborCond : Inport  Port=2
  Compare To Constant : simulink/Logic and Bit Operations/Compare To Constant  relop=~=  const=ModSts.ActiveState  OutDataTypeStr=boolean
  Compare To Constant1 : simulink/Logic and Bit Operations/Compare To Constant  relop===  const=FALSE  OutDataTypeStr=boolean
  Constant : Constant  Value=ModSts.StandbyState
  Switch : Switch  Criteria=u2 ~= 0
  Switch1 : Switch  Criteria=u2 ~= 0
  FCTBFuncImpArb : Outport
  wires:
  FCTBModStsArb/1 -> Switch1/1, Switch/1, Compare To Constant/1
  Compare To Constant/1 -> Switch/2
  FCTBFuncImpAborCond/1 -> Compare To Constant1/1
  Compare To Constant1/1 -> Switch1/2
  Constant/1 -> Switch1/3
  Switch1/1 -> Switch/3
  Switch/1 -> FCTBFuncImpArb/1

== system TosAeb/FcwAebJa_Step_sys/ALG_AEBSystem/ALG_AEBController/AEB_FuncImpManagementApp/FuncImpArbCalc/BrkFuncImpArbCalc1/BrkFuncImpArbCalc/JA_vehAEBfullFuncImpArbCalc ==
  JA_vehAEBfullfullModStsArb : Inport
  JA_vehAEBfullFuncImpAborCond : Inport  Port=2
  Compare To Constant : simulink/Logic and Bit Operations/Compare To Constant  relop=~=  const=ModSts.ActiveState  OutDataTypeStr=boolean
  Compare To Constant1 : simulink/Logic and Bit Operations/Compare To Constant  relop===  const=FALSE  OutDataTypeStr=boolean
  Constant : Constant  Value=ModSts.StandbyState
  Switch : Switch  Criteria=u2 ~= 0
  Switch1 : Switch  Criteria=u2 ~= 0
  JA_vehAEBfullFuncImpArb : Outport
  wires:
  Switch/1 -> JA_vehAEBfullFuncImpArb/1
  Switch1/1 -> Switch/3
  Constant/1 -> Switch1/3
  Compare To Constant1/1 -> Switch1/2
  JA_vehAEBfullFuncImpAborCond/1 -> Compare To Constant1/1
  Compare To Constant/1 -> Switch/2
  JA_vehAEBfullfullModStsArb/1 -> Compare To Constant/1, Switch/1, Switch1/1

== system TosAeb/FcwAebJa_Step_sys/ALG_AEBSystem/ALG_AEBController/AEB_FuncImpManagementApp/FuncImpArbCalc/BrkFuncImpArbCalc1/BrkFuncImpArbCalc/cycAEBfullFuncImpArbCalc ==
  cycAEBfullModStsArb : Inport
  cycAEBfullFuncImpAborCond : Inport  Port=2
  Compare To Constant : simulink/Logic and Bit Operations/Compare To Constant  relop=~=  const=ModSts.ActiveState  OutDataTypeStr=boolean
  Compare To Constant1 : simulink/Logic and Bit Operations/Compare To Constant  relop===  const=FALSE  OutDataTypeStr=boolean
  Constant : Constant  Value=ModSts.StandbyState
  Switch : Switch  Criteria=u2 ~= 0
  Switch1 : Switch  Criteria=u2 ~= 0
  cycAEBfullFuncImpArb : Outport
  wires:
  cycAEBfullModStsArb/1 -> Switch1/1, Switch/1, Compare To Constant/1
  Compare To Constant/1 -> Switch/2
  cycAEBfullFuncImpAborCond/1 -> Compare To Constant1/1
  Compare To Constant1/1 -> Switch1/2
  Constant/1 -> Switch1/3
  Switch1/1 -> Switch/3
  Switch/1 -> cycAEBfullFuncImpArb/1

== system TosAeb/FcwAebJa_Step_sys/ALG_AEBSystem/ALG_AEBController/AEB_FuncImpManagementApp/FuncImpArbCalc/BrkFuncImpArbCalc1/BrkFuncImpArbCalc/cycAEBpartFuncImpArbCalc ==
  cycAEBpartModStsArb : Inport
  cycAEBpartFuncImpAborCond : Inport  Port=2
  Compare To Constant : simulink/Logic and Bit Operations/Compare To Constant  relop=~=  const=ModSts.ActiveState  OutDataTypeStr=boolean
  Compare To Constant1 : simulink/Logic and Bit Operations/Compare To Constant  relop===  const=FALSE  OutDataTypeStr=boolean
  Constant : Constant  Value=ModSts.StandbyState
  Switch : Switch  Criteria=u2 ~= 0
  Switch1 : Switch  Criteria=u2 ~= 0
  cycAEBpartFuncImpArb : Outport
  wires:
  Switch/1 -> cycAEBpartFuncImpArb/1
  Switch1/1 -> Switch/3
  Constant/1 -> Switch1/3
  Compare To Constant1/1 -> Switch1/2
  cycAEBpartFuncImpAborCond/1 -> Compare To Constant1/1
  Compare To Constant/1 -> Switch/2
  cycAEBpartModStsArb/1 -> Compare To Constant/1, Switch/1, Switch1/1

== system TosAeb/FcwAebJa_Step_sys/ALG_AEBSystem/ALG_AEBController/AEB_FuncImpManagementApp/FuncImpArbCalc/BrkFuncImpArbCalc1/BrkFuncImpArbCalc/pedAEBfullFuncImpArbCalc ==
  pedAEBfullModStsArb : Inport
  pedAEBfullFuncImpAborCond : Inport  Port=2
  Compare To Constant : simulink/Logic and Bit Operations/Compare To Constant  relop=~=  const=ModSts.ActiveState  OutDataTypeStr=boolean
  Compare To Constant1 : simulink/Logic and Bit Operations/Compare To Constant  relop===  const=FALSE  OutDataTypeStr=boolean
  Constant : Constant  Value=ModSts.StandbyState
  Switch : Switch  Criteria=u2 ~= 0
  Switch1 : Switch  Criteria=u2 ~= 0
  pedAEBfullFuncImpArb : Outport
  wires:
  Switch/1 -> pedAEBfullFuncImpArb/1
  Switch1/1 -> Switch/3
  Constant/1 -> Switch1/3
  Compare To Constant1/1 -> Switch1/2
  pedAEBfullFuncImpAborCond/1 -> Compare To Constant1/1
  Compare To Constant/1 -> Switch/2
  pedAEBfullModStsArb/1 -> Compare To Constant/1, Switch/1, Switch1/1

== system TosAeb/FcwAebJa_Step_sys/ALG_AEBSystem/ALG_AEBController/AEB_FuncImpManagementApp/FuncImpArbCalc/BrkFuncImpArbCalc1/BrkFuncImpSuppCondCalc/AEBfullFuncImpSuppCondCalc ==
  AEBModStsArb : Inport
  Bus Selector4 : BusSelector  OutputSignals=latAEBModStsArb.pedAEBfullModStsArb,latAEBModStsArb.cycAEBfullModStsArb,vehAEBModStsArb.EBAModStsArb
  Compare To Constant23 : simulink/Logic and Bit Operations/Compare To Constant  relop===  const=ModSts.ActiveState  OutDataTypeStr=boolean
  Compare To Constant24 : simulink/Logic and Bit Operations/Compare To Constant  relop===  const=ModSts.ActiveState  OutDataTypeStr=boolean
  Compare To Constant25 : simulink/Logic and Bit Operations/Compare To Constant  relop===  const=ModSts.ActiveState  OutDataTypeStr=boolean
  Logical Operator4 : Logic  Operator=OR  Inputs=3  AllPortsSameDT=off  OutDataTypeStr=boolean
  AEBfullFuncImpSuppCond : Outport
  wires:
  Bus Selector4/2 -> Compare To Constant24/1  "<cycAEBfullModStsArb>"
  Bus Selector4/3 -> Compare To Constant25/1  "<EBAModStsArb>"
  Bus Selector4/1 -> Compare To Constant23/1  "<pedAEBfullModStsArb>"
  Logical Operator4/1 -> AEBfullFuncImpSuppCond/1
  Compare To Constant25/1 -> Logical Operator4/3
  Compare To Constant23/1 -> Logical Operator4/1
  Compare To Constant24/1 -> Logical Operator4/2
  AEBModStsArb/1 -> Bus Selector4/1

== system TosAeb/FcwAebJa_Step_sys/ALG_AEBSystem/ALG_AEBController/AEB_FuncImpManagementApp/FuncImpArbCalc/BrkFuncImpArbCalc1/BrkFuncImpSuppCondCalc/AEBpartFuncImpSuppCondCalc ==
  AEBModStsArb : Inport
  Bus Selector1 : BusSelector  OutputSignals=vehAEBModStsArb.AEBfullModStsArb,latAEBModStsArb.pedAEBfullModStsArb,latAEBModStsArb.cycAEBpartModStsArb,latAEBModStsArb.cycAEBfullModStsArb,JA_vehAEBModStsArb.JA_vehAEBfullModStsArb,vehAEBModStsArb.EBAModStsArb
  Compare To Constant{7..12} (6 alike) : simulink/Logic and Bit Operations/Compare To Constant  relop===  const=ModSts.ActiveState  OutDataTypeStr=boolean
  Logical Operator1 : Logic  Operator=OR  Inputs=6  AllPortsSameDT=off  OutDataTypeStr=boolean
  AEBpartFuncImpSuppCond : Outport
  wires:
  Bus Selector1/2 -> Compare To Constant8/1  "<pedAEBfullModStsArb>"
  Bus Selector1/4 -> Compare To Constant10/1  "<cycAEBfullModStsArb>"
  Bus Selector1/3 -> Compare To Constant9/1  "<cycAEBpartModStsArb>"
  Bus Selector1/1 -> Compare To Constant7/1  "<AEBfullModStsArb>"
  Bus Selector1/5 -> Compare To Constant11/1  "<JA_vehAEBfullModStsArb>"
  Compare To Constant9/1 -> Logical Operator1/3
  Compare To Constant10/1 -> Logical Operator1/4
  Compare To Constant8/1 -> Logical Operator1/2
  Compare To Constant11/1 -> Logical Operator1/5
  Compare To Constant7/1 -> Logical Operator1/1
  Compare To Constant12/1 -> Logical Operator1/6
  Logical Operator1/1 -> AEBpartFuncImpSuppCond/1
  Bus Selector1/6 -> Compare To Constant12/1  "<EBAModStsArb>"
  AEBModStsArb/1 -> Bus Selector1/1

== system TosAeb/FcwAebJa_Step_sys/ALG_AEBSystem/ALG_AEBController/AEB_FuncImpManagementApp/FuncImpArbCalc/BrkFuncImpArbCalc1/BrkFuncImpSuppCondCalc/EBAFuncImpSuppCondCalc ==
  Constant : Constant  Value=FALSE
  EBAFuncImpSuppCond : Outport
  wires:
  Constant/1 -> EBAFuncImpSuppCond/1

== system TosAeb/FcwAebJa_Step_sys/ALG_AEBSystem/ALG_AEBController/AEB_FuncImpManagementApp/FuncImpArbCalc/BrkFuncImpArbCalc1/BrkFuncImpSuppCondCalc/FCTBFuncImpSuppCondCalc ==
  AEBModStsArb : Inport
  Bus Selector : BusSelector  OutputSignals=vehAEBModStsArb.AEBpartModStsArb,vehAEBModStsArb.AEBfullModStsArb,vehAEBModStsArb.EBAModStsArb,latAEBModStsArb.pedAEBfullModStsArb,latAEBModStsArb.cycAEBfullModStsArb,latAEBModStsArb.cycAEBpartModStsArb,JA_vehAEBModStsArb.JA_vehAEBfullModStsArb
  Compare To Constant{,1..6} (7 alike) : simulink/Logic and Bit Operations/Compare To Constant  relop===  const=ModSts.ActiveState  OutDataTypeStr=boolean
  Logical Operator : Logic  Operator=OR  Inputs=7  AllPortsSameDT=off  OutDataTypeStr=boolean
  FCTBFuncImpSuppCond : Outport
  wires:
  Bus Selector/1 -> Compare To Constant/1  "<AEBpartModStsArb>"
  Bus Selector/2 -> Compare To Constant1/1  "<AEBfullModStsArb>"
  Compare To Constant2/1 -> Logical Operator/3
  Compare To Constant5/1 -> Logical Operator/6
  Logical Operator/1 -> FCTBFuncImpSuppCond/1
  Compare To Constant4/1 -> Logical Operator/5
  Compare To Constant6/1 -> Logical Operator/7
  Compare To Constant1/1 -> Logical Operator/2
  Compare To Constant/1 -> Logical Operator/1
  Compare To Constant3/1 -> Logical Operator/4
  Bus Selector/6 -> Compare To Constant5/1  "<cycAEBpartModStsArb>"
  Bus Selector/5 -> Compare To Constant4/1  "<cycAEBfullModStsArb>"
  Bus Selector/7 -> Compare To Constant6/1  "<JA_vehAEBfullModStsArb>"
  Bus Selector/4 -> Compare To Constant3/1  "<pedAEBfullModStsArb>"
  Bus Selector/3 -> Compare To Constant2/1  "<EBAModStsArb>"
  AEBModStsArb/1 -> Bus Selector/1

== system TosAeb/FcwAebJa_Step_sys/ALG_AEBSystem/ALG_AEBController/AEB_FuncImpManagementApp/FuncImpArbCalc/BrkFuncImpArbCalc1/BrkFuncImpSuppCondCalc/JA_vehAEBfullFuncImpSuppCondCalc ==
  AEBModStsArb : Inport
  Bus Selector3 : BusSelector  OutputSignals=vehAEBModStsArb.AEBfullModStsArb,latAEBModStsArb.cycAEBfullModStsArb,latAEBModStsArb.pedAEBfullModStsArb,vehAEBModStsArb.EBAModStsArb
  Compare To Constant{18..21} (4 alike) : simulink/Logic and Bit Operations/Compare To Constant  relop===  const=ModSts.ActiveState  OutDataTypeStr=boolean
  Logical Operator3 : Logic  Operator=OR  Inputs=4  AllPortsSameDT=off  OutDataTypeStr=boolean
  JA_vehAEBfullFuncImpSuppCond : Outport
  wires:
  AEBModStsArb/1 -> Bus Selector3/1
  Bus Selector3/1 -> Compare To Constant18/1  "<AEBfullModStsArb>"
  Bus Selector3/3 -> Compare To Constant20/1  "<pedAEBfullModStsArb>"
  Bus Selector3/4 -> Compare To Constant21/1  "<EBAModStsArb>"
  Bus Selector3/2 -> Compare To Constant19/1  "<cycAEBfullModStsArb>"
  Compare To Constant20/1 -> Logical Operator3/3
  Logical Operator3/1 -> JA_vehAEBfullFuncImpSuppCond/1
  Compare To Constant19/1 -> Logical Operator3/2
  Compare To Constant18/1 -> Logical Operator3/1
  Compare To Constant21/1 -> Logical Operator3/4

== system TosAeb/FcwAebJa_Step_sys/ALG_AEBSystem/ALG_AEBController/AEB_FuncImpManagementApp/FuncImpArbCalc/BrkFuncImpArbCalc1/BrkFuncImpSuppCondCalc/cycAEBfullFuncImpSuppCondCalc ==
  AEBModStsArb : Inport
  Bus Selector5 : BusSelector  OutputSignals=latAEBModStsArb.pedAEBfullModStsArb,vehAEBModStsArb.EBAModStsArb
  Compare To Constant22 : simulink/Logic and Bit Operations/Compare To Constant  relop===  const=ModSts.ActiveState  OutDataTypeStr=boolean
  Compare To Constant26 : simulink/Logic and Bit Operations/Compare To Constant  relop===  const=ModSts.ActiveState  OutDataTypeStr=boolean
  Logical Operator5 : Logic  Operator=OR  AllPortsSameDT=off  OutDataTypeStr=boolean
  cycAEBfullFuncImpSuppCond : Outport
  wires:
  Compare To Constant26/1 -> Logical Operator5/2
  Bus Selector5/1 -> Compare To Constant22/1  "<pedAEBfullModStsArb>"
  Logical Operator5/1 -> cycAEBfullFuncImpSuppCond/1
  Compare To Constant22/1 -> Logical Operator5/1
  Bus Selector5/2 -> Compare To Constant26/1  "<EBAModStsArb>"
  AEBModStsArb/1 -> Bus Selector5/1

== system TosAeb/FcwAebJa_Step_sys/ALG_AEBSystem/ALG_AEBController/AEB_FuncImpManagementApp/FuncImpArbCalc/BrkFuncImpArbCalc1/BrkFuncImpSuppCondCalc/cycAEBpartFuncImpSuppCondCalc ==
  AEBModStsArb : Inport
  Bus Selector2 : BusSelector  OutputSignals=vehAEBModStsArb.AEBfullModStsArb,latAEBModStsArb.pedAEBfullModStsArb,latAEBModStsArb.cycAEBfullModStsArb,JA_vehAEBModStsArb.JA_vehAEBfullModStsArb,vehAEBModStsArb.EBAModStsArb
  Compare To Constant{13..17} (5 alike) : simulink/Logic and Bit Operations/Compare To Constant  relop===  const=ModSts.ActiveState  OutDataTypeStr=boolean
  Logical Operator2 : Logic  Operator=OR  Inputs=5  AllPortsSameDT=off  OutDataTypeStr=boolean
  cycAEBpartFuncImpSuppCond : Outport
  wires:
  Bus Selector2/1 -> Compare To Constant13/1  "<AEBfullModStsArb>"
  Bus Selector2/3 -> Compare To Constant15/1  "<cycAEBfullModStsArb>"
  Bus Selector2/2 -> Compare To Constant14/1  "<pedAEBfullModStsArb>"
  Compare To Constant17/1 -> Logical Operator2/5
  Compare To Constant16/1 -> Logical Operator2/4
  Compare To Constant15/1 -> Logical Operator2/3
  Compare To Constant13/1 -> Logical Operator2/1
  Bus Selector2/4 -> Compare To Constant16/1  "<JA_vehAEBfullModStsArb>"
  Bus Selector2/5 -> Compare To Constant17/1  "<EBAModStsArb>"
  AEBModStsArb/1 -> Bus Selector2/1
  Logical Operator2/1 -> cycAEBpartFuncImpSuppCond/1
  Compare To Constant14/1 -> Logical Operator2/2

== system TosAeb/FcwAebJa_Step_sys/ALG_AEBSystem/ALG_AEBController/AEB_FuncImpManagementApp/FuncImpArbCalc/BrkFuncImpArbCalc1/BrkFuncImpSuppCondCalc/pedAEBfullFuncImpSuppCondCalc ==
  AEBModStsArb : Inport
  Bus Selector6 : BusSelector  OutputSignals=vehAEBModStsArb.EBAModStsArb
  Compare To Constant27 : simulink/Logic and Bit Operations/Compare To Constant  relop===  const=ModSts.ActiveState  OutDataTypeStr=boolean
  pedAEBfullFuncImpSuppCond : Outport
  wires:
  Bus Selector6/1 -> Compare To Constant27/1  "<EBAModStsArb>"
  Compare To Constant27/1 -> pedAEBfullFuncImpSuppCond/1
  AEBModStsArb/1 -> Bus Selector6/1

== system TosAeb/FcwAebJa_Step_sys/ALG_AEBSystem/ALG_AEBController/AEB_FuncImpManagementApp/FuncImpArbCalc/BrkReadyFuncImpArbCalc/BrkReadyFuncImpArbCalc/ABAFuncImpArbCalc ==
  ABAModStsArb : Inport
  ABAFuncImpAborCond : Inport  Port=2
  Compare To Constant : simulink/Logic and Bit Operations/Compare To Constant  relop=~=  const=ModSts.ActiveState  OutDataTypeStr=boolean
  Compare To Constant1 : simulink/Logic and Bit Operations/Compare To Constant  relop===  const=FALSE  OutDataTypeStr=boolean
  Constant : Constant  Value=ModSts.StandbyState
  Switch : Switch  Criteria=u2 ~= 0
  Switch1 : Switch  Criteria=u2 ~= 0
  ABAFuncImpArb : Outport
  wires:
  ABAModStsArb/1 -> Switch1/1, Switch/1, Compare To Constant/1
  Compare To Constant/1 -> Switch/2
  ABAFuncImpAborCond/1 -> Compare To Constant1/1
  Compare To Constant1/1 -> Switch1/2
  Constant/1 -> Switch1/3
  Switch1/1 -> Switch/3
  Switch/1 -> ABAFuncImpArb/1

== system TosAeb/FcwAebJa_Step_sys/ALG_AEBSystem/ALG_AEBController/AEB_FuncImpManagementApp/FuncImpArbCalc/BrkReadyFuncImpArbCalc/BrkReadyFuncImpArbCalc/FCTB_ABAFuncImpArbCalc ==
  FCTB_ABAModStsArb : Inport
  FCTB_ABAFuncImpAborCond : Inport  Port=2
  Compare To Constant : simulink/Logic and Bit Operations/Compare To Constant  relop=~=  const=ModSts.ActiveState  OutDataTypeStr=boolean
  Compare To Constant1 : simulink/Logic and Bit Operations/Compare To Constant  relop===  const=FALSE  OutDataTypeStr=boolean
  Constant : Constant  Value=ModSts.StandbyState
  Switch : Switch  Criteria=u2 ~= 0
  Switch1 : Switch  Criteria=u2 ~= 0
  FCTB_ABAFuncImpArb : Outport
  wires:
  Switch/1 -> FCTB_ABAFuncImpArb/1
  Switch1/1 -> Switch/3
  Constant/1 -> Switch1/3
  Compare To Constant1/1 -> Switch1/2
  FCTB_ABAFuncImpAborCond/1 -> Compare To Constant1/1
  Compare To Constant/1 -> Switch/2
  FCTB_ABAModStsArb/1 -> Compare To Constant/1, Switch/1, Switch1/1
